simulink model "V2x_TC_IntelligentTrafficSigns_cs"  (Simulink 7.6)
settings: Solver=FixedStepDiscrete  SolverMode=SingleTasking  StartTime=0.0  StopTime=66  FixedStep=1/100  RelTol=1e-3
PreLoadFcn:
  | check_NUTS_defined
  | 
  | assignin('base',['model_closed_' bdroot],false);addpath('../includes_V2x');
InitFcn:
  | PreScanExperimentInitFcn(bdroot)
StopFcn:
  | PreScanExperimentStopFcn(bdroot);
  | 
undefined symbols (not defined in the file or in its folder): acceleration, actorID, actorType, DiscIntNeverNeededParam, GPS_alt, GPS_lat, GPS_long, lightBarInUse, messageID, Newton, publicActorType, Raphson, rotZ, sigbuilder_block, sireneInUse, trafficLightPriority, turnAdvice, velocity, x_ini_VRML, y_ini_VRML, yawRate, z_ini_VRML
libraries: simulink x111, PreScanSimulinkLibrary x7, simulink_extras x4, PreScanUsefulBlocks x3
(part 2 of 16)

== system V2x_TC_IntelligentTrafficSigns_cs/CameraTrolley ==
  SELF_CameraTrolley : Inport  Tag=SELFInport
  Dynamics_Empty : Subsystem  Tag=Dynamics_Empty
      mask Display: port_label('output', 1, 'State');text(0,0,' Empty\n')
  SELF_Demux : Subsystem  Tag=DemuxSELF
      settings: DeleteFcn=PreScanBlockDeleteFcn;
      mask Type: PreScan Demux Block:
      mask Display:
      | port_label('output',1,'x [m]')
      | port_label('output',2,'y [m]')
      | port_label('output',3,'z [m]','texmode','on')
      | port_label('output',4,'Rotx [deg]','texmode','on')
      | port_label('output',5,'Roty [deg]','texmode','on')
      | port_label('output',6,'Rotz [deg]','texmode','on')
      | port_label('output',7,'GPS lat [deg]','texmode','on')
      | port_label('output',8,'GPS long [deg]','texmode','on')
      | port_label('output',9,'GPS alt [m]','texmode','on')
      | port_label('output',10,'Velocity [m/s]')
      | port_label('output',11,'Heading [deg]')
      | port_label('output',12,'Yaw rate [deg/s]')
  Terminator_{1..13} (13 alike) : Terminator
  transmits only position : Gain  Gain=[1 1 1 0 0 0 0 0 1]
  STATE_CameraTrolley : Outport  Tag=StateOutport
  wires:
  [State_BMW] -> transmits only position/1
  SELF_CameraTrolley/1 -> SELF_Demux/1(SELF)
  transmits only position/1 -> STATE_CameraTrolley/1
  Dynamics_Empty/1(State) -> Terminator_1/1
  SELF_Demux/1(x [m]) -> Terminator_2/1
  SELF_Demux/2(y [m]) -> Terminator_3/1
  SELF_Demux/3(z [m]) -> Terminator_4/1
  SELF_Demux/4(rotx [deg]) -> Terminator_5/1
  SELF_Demux/5(roty [deg]) -> Terminator_6/1
  SELF_Demux/6(rotz [deg]) -> Terminator_7/1
  SELF_Demux/7(GPS lat [deg]) -> Terminator_8/1
  SELF_Demux/8(GPS long [deg]) -> Terminator_9/1
  SELF_Demux/9(GPS alt [m]) -> Terminator_10/1
  SELF_Demux/10(velocity [m/s]) -> Terminator_11/1
  SELF_Demux/11(heading [deg]) -> Terminator_12/1
  SELF_Demux/12(yaw rate [deg/s]) -> Terminator_13/1

== system V2x_TC_IntelligentTrafficSigns_cs/Car_Rx_1 ==
  Selector : Selector  IndexMode=Zero-based  IndexOptions=Starting index (dialog)  Indices=PortIndex  OutputSizes=PortWidth(1)
  U : Outport
  wires:
  Selector/1 -> U/1
  [SimCoreSensors] -> Selector/1

== system V2x_TC_IntelligentTrafficSigns_cs/Car_Tx_1 ==
  Y : Inport
  Assignment : Assignment  IndexMode=Zero-based  SampleTime=TimeStep  IndexOptions=Starting index (dialog)  Indices=PortIndex  OutputSizes=[1 1]
  Data Store Read : DataStoreRead  DataStoreName=SimCoreActuators  SampleTime=TimeStep
  Data Store Write : DataStoreWrite  DataStoreName=SimCoreActuators
  wires:
  Assignment/1 -> Data Store Write/1
  Data Store Read/1 -> Assignment/1
  Y/1 -> Assignment/2

== system V2x_TC_IntelligentTrafficSigns_cs/GetSensors ==
  dummy : Inport
  GetSensors : M-S-Function  FunctionName=GetSensorsBlock  Parameters=TimeStep NrOfSensors
  tag scope: SimCoreSensors=global
  wires:
  GetSensors/1 -> [SimCoreSensors]
  dummy/1 -> GetSensors/1

== system V2x_TC_IntelligentTrafficSigns_cs/Noise generator ==
  DISPLAY : M-S-Function  FunctionName=msfcn_V2X_PlotSpeed
  Scope1 : Scope  NumInputPorts=4
      settings: YMin=-5~-5~-5~-5  YMax=5~5~5~5
  tag scope: NMM_Noise=global, Tdelay=global
  wires:
  DISPLAY/1 -> Scope1/1
  DISPLAY/2 -> Scope1/2
  DISPLAY/3 -> Scope1/3, [NMM_Noise]
  DISPLAY/4 -> Scope1/4, [Tdelay]

== system V2x_TC_IntelligentTrafficSigns_cs/SELF_BMW_X5_1 ==
  Selector : Selector  IndexMode=Zero-based  IndexOptions=Starting index (dialog)  Indices=PortIndex  OutputSizes=PortWidth(1)
  U : Outport
  wires:
  Selector/1 -> U/1
  [SimCoreSensors] -> Selector/1

== system V2x_TC_IntelligentTrafficSigns_cs/SELF_CameraTrolley ==
  Selector : Selector  IndexMode=Zero-based  IndexOptions=Starting index (dialog)  Indices=PortIndex  OutputSizes=PortWidth(1)
  U : Outport
  wires:
  Selector/1 -> U/1
  [SimCoreSensors] -> Selector/1

== system V2x_TC_IntelligentTrafficSigns_cs/STATE_BMW_X5_1 ==
  State : Inport  SampleTime=TimeStep
  Acceleration : Subsystem  mask(ToSensorWorld){PortName=Acceleration_BMW_X5_1, PortIndex=6, PortWidth=3, TimeStep=1/100}
      mask prompts: PortName "Port Name [-]"; PortIndex "Index [-]"; PortWidth "Port Dimensions [-]"; TimeStep "Upload Period [s]"
      mask help: Sends signal(s) from Simulink to the Sensor World
      mask Help:
      | Sends signal(s) from Simulink to the Sensor World.
      | 
      | - Port Name [-]: specifies the name of the output terminal of the Simulink block.
      | - Index [-]: specifies the start position in the output vector sent to the Sensor World
      | - Port dimensions [-]: specifies the width (number of scalars) of the output port
      | - Upload period [s]: specifies the upload period (in seconds) of the port.
      mask Display: port_label('input', 1, PortName)
  Demux : Demux  Outputs=5
  Position : Subsystem  mask(ToSensorWorld){PortName=Position_BMW_X5_1, PortIndex=0, PortWidth=3, TimeStep=1/100}
      mask prompts: PortName "Port Name [-]"; PortIndex "Index [-]"; PortWidth "Port Dimensions [-]"; TimeStep "Upload Period [s]"
      mask help: Sends signal(s) from Simulink to the Sensor World
      mask Help:
      | Sends signal(s) from Simulink to the Sensor World.
      | 
      | - Port Name [-]: specifies the name of the output terminal of the Simulink block.
      | - Index [-]: specifies the start position in the output vector sent to the Sensor World
      | - Port dimensions [-]: specifies the width (number of scalars) of the output port
      | - Upload period [s]: specifies the upload period (in seconds) of the port.
      mask Display: port_label('input', 1, PortName)
  RPY : Subsystem  mask(ToSensorWorld){PortName=RPY_BMW_X5_1, PortIndex=9, PortWidth=3, TimeStep=1/100}
      mask prompts: PortName "Port Name [-]"; PortIndex "Index [-]"; PortWidth "Port Dimensions [-]"; TimeStep "Upload Period [s]"
      mask help: Sends signal(s) from Simulink to the Sensor World
      mask Help:
      | Sends signal(s) from Simulink to the Sensor World.
      | 
      | - Port Name [-]: specifies the name of the output terminal of the Simulink block.
      | - Index [-]: specifies the start position in the output vector sent to the Sensor World
      | - Port dimensions [-]: specifies the width (number of scalars) of the output port
      | - Upload period [s]: specifies the upload period (in seconds) of the port.
      mask Display: port_label('input', 1, PortName)
  Velocity : Subsystem  mask(ToSensorWorld){PortName=Velocity_BMW_X5_1, PortIndex=3, PortWidth=3, TimeStep=1/100}
      mask prompts: PortName "Port Name [-]"; PortIndex "Index [-]"; PortWidth "Port Dimensions [-]"; TimeStep "Upload Period [s]"
      mask help: Sends signal(s) from Simulink to the Sensor World
      mask Help:
      | Sends signal(s) from Simulink to the Sensor World.
      | 
      | - Port Name [-]: specifies the name of the output terminal of the Simulink block.
      | - Index [-]: specifies the start position in the output vector sent to the Sensor World
      | - Port dimensions [-]: specifies the width (number of scalars) of the output port
      | - Upload period [s]: specifies the upload period (in seconds) of the port.
      mask Display: port_label('input', 1, PortName)
  iniBODYAngles wrtVEH [rad] : Constant  Value=[Roll0 Pitch0 0]  SampleTime=TimeStep
  iniVEHAngles wrtGLOB [rad] : Constant  Value=[0 0 Yaw0]  SampleTime=TimeStep
  iniVEHposition wrtGLOB [m] : Constant  Value=[x0 y0 z0]  SampleTime=TimeStep
  state2FullState : PreScanSimulinkLibrary/state2FullState  SystemSampleTime=-1
  velRPY : Subsystem  mask(ToSensorWorld){PortName=velRPY_BMW_X5_1, PortIndex=12, PortWidth=3, TimeStep=1/100}
      mask prompts: PortName "Port Name [-]"; PortIndex "Index [-]"; PortWidth "Port Dimensions [-]"; TimeStep "Upload Period [s]"
      mask help: Sends signal(s) from Simulink to the Sensor World
      mask Help:
      | Sends signal(s) from Simulink to the Sensor World.
      | 
      | - Port Name [-]: specifies the name of the output terminal of the Simulink block.
      | - Index [-]: specifies the start position in the output vector sent to the Sensor World
      | - Port dimensions [-]: specifies the width (number of scalars) of the output port
      | - Upload period [s]: specifies the upload period (in seconds) of the port.
      mask Display: port_label('input', 1, PortName)
  wires:
  Demux/5 -> velRPY/1(Y)
  Demux/4 -> RPY/1(Y)
  Demux/3 -> Acceleration/1(Y)
  Demux/2 -> Velocity/1(Y)
  Demux/1 -> Position/1(Y)
  State/1 -> state2FullState/1
  state2FullState/1 -> Demux/1
  iniVEHposition wrtGLOB [m]/1 -> state2FullState/2
  iniBODYAngles wrtVEH [rad]/1 -> state2FullState/3
  iniVEHAngles wrtGLOB [rad]/1 -> state2FullState/4

== system V2x_TC_IntelligentTrafficSigns_cs/STATE_CameraTrolley ==
  State : Inport  SampleTime=TimeStep
  Acceleration : Subsystem  mask(ToSensorWorld){PortName=Acceleration_CameraTrolley, PortIndex=454, PortWidth=3, TimeStep=1/100}
      mask prompts: PortName "Port Name [-]"; PortIndex "Index [-]"; PortWidth "Port Dimensions [-]"; TimeStep "Upload Period [s]"
      mask help: Sends signal(s) from Simulink to the Sensor World
      mask Help:
      | Sends signal(s) from Simulink to the Sensor World.
      | 
      | - Port Name [-]: specifies the name of the output terminal of the Simulink block.
      | - Index [-]: specifies the start position in the output vector sent to the Sensor World
      | - Port dimensions [-]: specifies the width (number of scalars) of the output port
      | - Upload period [s]: specifies the upload period (in seconds) of the port.
      mask Display: port_label('input', 1, PortName)
  Demux : Demux  Outputs=5
  Position : Subsystem  mask(ToSensorWorld){PortName=Position_CameraTrolley, PortIndex=448, PortWidth=3, TimeStep=1/100}
      mask prompts: PortName "Port Name [-]"; PortIndex "Index [-]"; PortWidth "Port Dimensions [-]"; TimeStep "Upload Period [s]"
      mask help: Sends signal(s) from Simulink to the Sensor World
      mask Help:
      | Sends signal(s) from Simulink to the Sensor World.
      | 
      | - Port Name [-]: specifies the name of the output terminal of the Simulink block.
      | - Index [-]: specifies the start position in the output vector sent to the Sensor World
      | - Port dimensions [-]: specifies the width (number of scalars) of the output port
      | - Upload period [s]: specifies the upload period (in seconds) of the port.
      mask Display: port_label('input', 1, PortName)
  RPY : Subsystem  mask(ToSensorWorld){PortName=RPY_CameraTrolley, PortIndex=457, PortWidth=3, TimeStep=1/100}
      mask prompts: PortName "Port Name [-]"; PortIndex "Index [-]"; PortWidth "Port Dimensions [-]"; TimeStep "Upload Period [s]"
      mask help: Sends signal(s) from Simulink to the Sensor World
      mask Help:
      | Sends signal(s) from Simulink to the Sensor World.
      | 
      | - Port Name [-]: specifies the name of the output terminal of the Simulink block.
      | - Index [-]: specifies the start position in the output vector sent to the Sensor World
      | - Port dimensions [-]: specifies the width (number of scalars) of the output port
      | - Upload period [s]: specifies the upload period (in seconds) of the port.
      mask Display: port_label('input', 1, PortName)
  Velocity : Subsystem  mask(ToSensorWorld){PortName=Velocity_CameraTrolley, PortIndex=451, PortWidth=3, TimeStep=1/100}
      mask prompts: PortName "Port Name [-]"; PortIndex "Index [-]"; PortWidth "Port Dimensions [-]"; TimeStep "Upload Period [s]"
      mask help: Sends signal(s) from Simulink to the Sensor World
      mask Help:
      | Sends signal(s) from Simulink to the Sensor World.
      | 
      | - Port Name [-]: specifies the name of the output terminal of the Simulink block.
      | - Index [-]: specifies the start position in the output vector sent to the Sensor World
      | - Port dimensions [-]: specifies the width (number of scalars) of the output port
      | - Upload period [s]: specifies the upload period (in seconds) of the port.
      mask Display: port_label('input', 1, PortName)
  iniBODYAngles wrtVEH [rad] : Constant  Value=[Roll0 Pitch0 0]  SampleTime=TimeStep
  iniVEHAngles wrtGLOB [rad] : Constant  Value=[0 0 Yaw0]  SampleTime=TimeStep
  iniVEHposition wrtGLOB [m] : Constant  Value=[x0 y0 z0]  SampleTime=TimeStep
  state2FullState : PreScanSimulinkLibrary/state2FullState  SystemSampleTime=-1
  velRPY : Subsystem  mask(ToSensorWorld){PortName=velRPY_CameraTrolley, PortIndex=460, PortWidth=3, TimeStep=1/100}
      mask prompts: PortName "Port Name [-]"; PortIndex "Index [-]"; PortWidth "Port Dimensions [-]"; TimeStep "Upload Period [s]"
      mask help: Sends signal(s) from Simulink to the Sensor World
      mask Help:
      | Sends signal(s) from Simulink to the Sensor World.
      | 
      | - Port Name [-]: specifies the name of the output terminal of the Simulink block.
      | - Index [-]: specifies the start position in the output vector sent to the Sensor World
      | - Port dimensions [-]: specifies the width (number of scalars) of the output port
      | - Upload period [s]: specifies the upload period (in seconds) of the port.
      mask Display: port_label('input', 1, PortName)
  wires:
  Demux/5 -> velRPY/1(Y)
  Demux/4 -> RPY/1(Y)
  Demux/3 -> Acceleration/1(Y)
  Demux/2 -> Velocity/1(Y)
  Demux/1 -> Position/1(Y)
  State/1 -> state2FullState/1
  state2FullState/1 -> Demux/1
  iniVEHposition wrtGLOB [m]/1 -> state2FullState/2
  iniBODYAngles wrtVEH [rad]/1 -> state2FullState/3
  iniVEHAngles wrtGLOB [rad]/1 -> state2FullState/4

== system V2x_TC_IntelligentTrafficSigns_cs/SetActuators ==
  Bus Creator : BusCreator  Inputs=1
  Data Store Read : DataStoreRead  DataStoreName=SimCoreActuators  SampleTime=TimeStep
  SendActuators : M-S-Function  FunctionName=SendActuatorsBlock  Parameters=TimeStep NrOfActuators
  dummy : Outport
  tag scope: SimCoreInfo=global
  wires:
  Bus Creator/1 -> [SimCoreInfo]  "SimCore"
  SendActuators/2 -> Bus Creator/1  "ComputationTime[s]"
  SendActuators/1 -> dummy/1
  Data Store Read/1 -> SendActuators/1

== system V2x_TC_IntelligentTrafficSigns_cs/SimCoreInfo ==
  Bus Selector : BusSelector  OutputSignals=ComputationTime[s]
  Time : Scope
      settings: TimeRange=10  YMin=0  YMax=0.5  SaveName=ComputationTime
  wires:
  Bus Selector/1 -> Time/1  "<ComputationTime[s]>"
  [SimCoreInfo] -> Bus Selector/1

== system V2x_TC_IntelligentTrafficSigns_cs/TS_Lcurve_R14 ==
  CAM generation time step : Constant  Value=0.1
  CAM repetition time step : Constant  Value=0.05
  Cartesian to GPS : Subsystem
  Green light : Constant  Value=0
  Ground_{1..5} (5 alike) : Ground
  Infrastructure CAM generator : Subsystem
  Orange light : Constant  Value=0
  Red light : Constant  Value=0
  Tx_Lcurve_R14_BusAssignment : BusAssignment  Tag=BusAntennaT  AssignedSignals=warning,messageID,actorID,actorType,trafficLightPriority,GPS_lat,GPS_long,GPS_alt,rotZ
  Tx_Lcurve_R14_Mux : Subsystem  Tag=MuxAntennaT  mask(PreScan Mux Block:){Channel=1, TimeStep=1/100}
      mask prompts: TimeStep "Time Step [s]"
      settings: DeleteFcn=PreScanBlockDeleteFcn;
      mask Display:
      | fprintf('Channel: %d',Channel)
      | port_label('input', 1, 'Warning signal ID')
      | port_label('input', 2, 'Maneuvre ID')
      | port_label('input', 3, 'Vehicle Type')
      | port_label('input', 4, 'Braking Capacity')
      | port_label('input', 5, 'Vehicle Size')
      | port_label('input', 6, 'spare')
  X : Constant  Value=-192.331085
  Y : Constant  Value=-63.62283
  Z : Constant  Value=0
  actorID : Constant  Value=32
  actorType : Constant  Value=14
  messageID : Constant  Value=902
  rotZ : Constant  Value=90
  warning : Constant  Value=0
  Tx_Lcurve_R14 : Outport  Tag=AntennaTOutport
  wires:
  CAM generation time step/1 -> Infrastructure CAM generator/1(CAM gen time step)
  CAM repetition time step/1 -> Infrastructure CAM generator/2(CAM rep time step)
  warning/1 -> Infrastructure CAM generator/3(warning)
  messageID/1 -> Infrastructure CAM generator/4(messageID)
  actorID/1 -> Infrastructure CAM generator/5(actorID)
  actorType/1 -> Infrastructure CAM generator/6(actorType)
  Red light/1 -> Infrastructure CAM generator/7(Red light)
  Orange light/1 -> Infrastructure CAM generator/8(Orange light)
  Green light/1 -> Infrastructure CAM generator/9(Green light)
  rotZ/1 -> Infrastructure CAM generator/13(rotZ)
  X/1 -> Cartesian to GPS/1(X)
  Y/1 -> Cartesian to GPS/2(Y)
  Z/1 -> Cartesian to GPS/3(Z)
  Cartesian to GPS/1(lat) -> Infrastructure CAM generator/10(GPS_lat)
  Cartesian to GPS/2(long) -> Infrastructure CAM generator/11(GPS_long)
  Cartesian to GPS/3(alt) -> Infrastructure CAM generator/12(GPS_alt)
  Tx_Lcurve_R14_Mux/2(Out2) -> [Tx_Lcurve_R14]
  [Tx_Lcurve_R14] -> Tx_Lcurve_R14_BusAssignment/1
  Tx_Lcurve_R14_BusAssignment/1 -> Tx_Lcurve_R14_Mux/6(spare)
  Tx_Lcurve_R14_Mux/1(Out1) -> Tx_Lcurve_R14/1
  Infrastructure CAM generator/9(CAM_rotZ) -> Tx_Lcurve_R14_BusAssignment/10
  Infrastructure CAM generator/8(CAM_GPSalt) -> Tx_Lcurve_R14_BusAssignment/9
  Infrastructure CAM generator/7(CAM_GPSlong) -> Tx_Lcurve_R14_BusAssignment/8
  Infrastructure CAM generator/6(CAM_GPSlat) -> Tx_Lcurve_R14_BusAssignment/7
  Infrastructure CAM generator/5(CAM_trafficLightPriority) -> Tx_Lcurve_R14_BusAssignment/6
  Infrastructure CAM generator/4(CAM_vehicleType) -> Tx_Lcurve_R14_BusAssignment/5
  Infrastructure CAM generator/3(CAM_vehicleID) -> Tx_Lcurve_R14_BusAssignment/4
  Infrastructure CAM generator/2(CAM_messageID) -> Tx_Lcurve_R14_BusAssignment/3
  Infrastructure CAM generator/1(CAM_warning) -> Tx_Lcurve_R14_BusAssignment/2
  Ground_1/1 -> Tx_Lcurve_R14_Mux/1(warning_signal_id)
  Ground_2/1 -> Tx_Lcurve_R14_Mux/2(maneuvre_id)
  Ground_3/1 -> Tx_Lcurve_R14_Mux/3(vehicle_type)
  Ground_4/1 -> Tx_Lcurve_R14_Mux/4(brakingcapacity)
  Ground_5/1 -> Tx_Lcurve_R14_Mux/5(vehicle_size)

== system V2x_TC_IntelligentTrafficSigns_cs/TS_Lcurve_R25 ==
  CAM generation time step : Constant  Value=0.1
  CAM repetition time step : Constant  Value=0.05
  Cartesian to GPS : Subsystem
  Green light : Constant  Value=0
  Ground_{1..5} (5 alike) : Ground
  Infrastructure CAM generator : Subsystem
  Orange light : Constant  Value=0
  Red light : Constant  Value=0
  Tx_Lcurve_R25_BusAssignment : BusAssignment  Tag=BusAntennaT  AssignedSignals=warning,messageID,actorID,actorType,trafficLightPriority,GPS_lat,GPS_long,GPS_alt,rotZ
  Tx_Lcurve_R25_Mux : Subsystem  Tag=MuxAntennaT  mask(PreScan Mux Block:){Channel=1, TimeStep=1/100}
      mask prompts: TimeStep "Time Step [s]"
      settings: DeleteFcn=PreScanBlockDeleteFcn;
      mask Display:
      | fprintf('Channel: %d',Channel)
      | port_label('input', 1, 'Warning signal ID')
      | port_label('input', 2, 'Maneuvre ID')
      | port_label('input', 3, 'Vehicle Type')
      | port_label('input', 4, 'Braking Capacity')
      | port_label('input', 5, 'Vehicle Size')
      | port_label('input', 6, 'spare')
  X : Constant  Value=-105.98568
  Y : Constant  Value=-53.2315674
  Z : Constant  Value=0
  actorID : Constant  Value=902
  actorType : Constant  Value=14
  messageID : Constant  Value=902
  rotZ : Constant  Value=280
  warning : Constant  Value=0
  Tx_Lcurve_R25 : Outport  Tag=AntennaTOutport
  wires:
  CAM generation time step/1 -> Infrastructure CAM generator/1(CAM gen time step)
  CAM repetition time step/1 -> Infrastructure CAM generator/2(CAM rep time step)
  warning/1 -> Infrastructure CAM generator/3(warning)
  messageID/1 -> Infrastructure CAM generator/4(messageID)
  actorID/1 -> Infrastructure CAM generator/5(actorID)
  actorType/1 -> Infrastructure CAM generator/6(actorType)
  Red light/1 -> Infrastructure CAM generator/7(Red light)
  Orange light/1 -> Infrastructure CAM generator/8(Orange light)
  Green light/1 -> Infrastructure CAM generator/9(Green light)
  rotZ/1 -> Infrastructure CAM generator/13(rotZ)
  X/1 -> Cartesian to GPS/1(X)
  Y/1 -> Cartesian to GPS/2(Y)
  Z/1 -> Cartesian to GPS/3(Z)
  Cartesian to GPS/1(lat) -> Infrastructure CAM generator/10(GPS_lat)
  Cartesian to GPS/2(long) -> Infrastructure CAM generator/11(GPS_long)
  Cartesian to GPS/3(alt) -> Infrastructure CAM generator/12(GPS_alt)
  Tx_Lcurve_R25_Mux/2(Out2) -> [Tx_Lcurve_R25]
  [Tx_Lcurve_R25] -> Tx_Lcurve_R25_BusAssignment/1
  Tx_Lcurve_R25_BusAssignment/1 -> Tx_Lcurve_R25_Mux/6(spare)
  Tx_Lcurve_R25_Mux/1(Out1) -> Tx_Lcurve_R25/1
  Infrastructure CAM generator/9(CAM_rotZ) -> Tx_Lcurve_R25_BusAssignment/10
  Infrastructure CAM generator/8(CAM_GPSalt) -> Tx_Lcurve_R25_BusAssignment/9
  Infrastructure CAM generator/7(CAM_GPSlong) -> Tx_Lcurve_R25_BusAssignment/8
  Infrastructure CAM generator/6(CAM_GPSlat) -> Tx_Lcurve_R25_BusAssignment/7
  Infrastructure CAM generator/5(CAM_trafficLightPriority) -> Tx_Lcurve_R25_BusAssignment/6
  Infrastructure CAM generator/4(CAM_vehicleType) -> Tx_Lcurve_R25_BusAssignment/5
  Infrastructure CAM generator/3(CAM_vehicleID) -> Tx_Lcurve_R25_BusAssignment/4
  Infrastructure CAM generator/2(CAM_messageID) -> Tx_Lcurve_R25_BusAssignment/3
  Infrastructure CAM generator/1(CAM_warning) -> Tx_Lcurve_R25_BusAssignment/2
  Ground_1/1 -> Tx_Lcurve_R25_Mux/1(warning_signal_id)
  Ground_2/1 -> Tx_Lcurve_R25_Mux/2(maneuvre_id)
  Ground_3/1 -> Tx_Lcurve_R25_Mux/3(vehicle_type)
  Ground_4/1 -> Tx_Lcurve_R25_Mux/4(brakingcapacity)
  Ground_5/1 -> Tx_Lcurve_R25_Mux/5(vehicle_size)

== system V2x_TC_IntelligentTrafficSigns_cs/TS_Lcurve_R39 ==
  CAM generation time step : Constant  Value=0.1
  CAM repetition time step : Constant  Value=0.05
  Cartesian to GPS : Subsystem
  Green light : Constant  Value=0
  Ground_{1..5} (5 alike) : Ground
  Infrastructure CAM generator : Subsystem
  Orange light : Constant  Value=0
  Red light : Constant  Value=0
  Tx_Lcurve_R39_BusAssignment : BusAssignment  Tag=BusAntennaT  AssignedSignals=warning,messageID,actorID,actorType,trafficLightPriority,GPS_lat,GPS_long,GPS_alt,rotZ
  Tx_Lcurve_R39_Mux : Subsystem  Tag=MuxAntennaT  mask(PreScan Mux Block:){Channel=1, TimeStep=1/100}
      mask prompts: TimeStep "Time Step [s]"
      settings: DeleteFcn=PreScanBlockDeleteFcn;
      mask Display:
      | fprintf('Channel: %d',Channel)
      | port_label('input', 1, 'Warning signal ID')
      | port_label('input', 2, 'Maneuvre ID')
      | port_label('input', 3, 'Vehicle Type')
      | port_label('input', 4, 'Braking Capacity')
      | port_label('input', 5, 'Vehicle Size')
      | port_label('input', 6, 'spare')
  X : Constant  Value=-115.560738
  Y : Constant  Value=-55.21178
  Z : Constant  Value=0
  actorID : Constant  Value=29
  actorType : Constant  Value=14
  messageID : Constant  Value=902
  rotZ : Constant  Value=100
  warning : Constant  Value=0
  Tx_Lcurve_R39 : Outport  Tag=AntennaTOutport
  wires:
  CAM generation time step/1 -> Infrastructure CAM generator/1(CAM gen time step)
  CAM repetition time step/1 -> Infrastructure CAM generator/2(CAM rep time step)
  warning/1 -> Infrastructure CAM generator/3(warning)
  messageID/1 -> Infrastructure CAM generator/4(messageID)
  actorID/1 -> Infrastructure CAM generator/5(actorID)
  actorType/1 -> Infrastructure CAM generator/6(actorType)
  Red light/1 -> Infrastructure CAM generator/7(Red light)
  Orange light/1 -> Infrastructure CAM generator/8(Orange light)
  Green light/1 -> Infrastructure CAM generator/9(Green light)
  rotZ/1 -> Infrastructure CAM generator/13(rotZ)
  X/1 -> Cartesian to GPS/1(X)
  Y/1 -> Cartesian to GPS/2(Y)
  Z/1 -> Cartesian to GPS/3(Z)
  Cartesian to GPS/1(lat) -> Infrastructure CAM generator/10(GPS_lat)
  Cartesian to GPS/2(long) -> Infrastructure CAM generator/11(GPS_long)
  Cartesian to GPS/3(alt) -> Infrastructure CAM generator/12(GPS_alt)
  Tx_Lcurve_R39_Mux/2(Out2) -> [Tx_Lcurve_R39]
  [Tx_Lcurve_R39] -> Tx_Lcurve_R39_BusAssignment/1
  Tx_Lcurve_R39_BusAssignment/1 -> Tx_Lcurve_R39_Mux/6(spare)
  Tx_Lcurve_R39_Mux/1(Out1) -> Tx_Lcurve_R39/1
  Infrastructure CAM generator/9(CAM_rotZ) -> Tx_Lcurve_R39_BusAssignment/10
  Infrastructure CAM generator/8(CAM_GPSalt) -> Tx_Lcurve_R39_BusAssignment/9
  Infrastructure CAM generator/7(CAM_GPSlong) -> Tx_Lcurve_R39_BusAssignment/8
  Infrastructure CAM generator/6(CAM_GPSlat) -> Tx_Lcurve_R39_BusAssignment/7
  Infrastructure CAM generator/5(CAM_trafficLightPriority) -> Tx_Lcurve_R39_BusAssignment/6
  Infrastructure CAM generator/4(CAM_vehicleType) -> Tx_Lcurve_R39_BusAssignment/5
  Infrastructure CAM generator/3(CAM_vehicleID) -> Tx_Lcurve_R39_BusAssignment/4
  Infrastructure CAM generator/2(CAM_messageID) -> Tx_Lcurve_R39_BusAssignment/3
  Infrastructure CAM generator/1(CAM_warning) -> Tx_Lcurve_R39_BusAssignment/2
  Ground_1/1 -> Tx_Lcurve_R39_Mux/1(warning_signal_id)
  Ground_2/1 -> Tx_Lcurve_R39_Mux/2(maneuvre_id)
  Ground_3/1 -> Tx_Lcurve_R39_Mux/3(vehicle_type)
  Ground_4/1 -> Tx_Lcurve_R39_Mux/4(brakingcapacity)
  Ground_5/1 -> Tx_Lcurve_R39_Mux/5(vehicle_size)

== system V2x_TC_IntelligentTrafficSigns_cs/TS_Lcurve_R55 ==
  CAM generation time step : Constant  Value=0.1
  CAM repetition time step : Constant  Value=0.05
  Cartesian to GPS : Subsystem
  Green light : Constant  Value=0
  Ground_{1..5} (5 alike) : Ground
  Infrastructure CAM generator : Subsystem
  Orange light : Constant  Value=0
  Red light : Constant  Value=0
  Tx_Lcurve_R55_BusAssignment : BusAssignment  Tag=BusAntennaT  AssignedSignals=warning,messageID,actorID,actorType,trafficLightPriority,GPS_lat,GPS_long,GPS_alt,rotZ
  Tx_Lcurve_R55_Mux : Subsystem  Tag=MuxAntennaT  mask(PreScan Mux Block:){Channel=1, TimeStep=1/100}
      mask prompts: TimeStep "Time Step [s]"
      settings: DeleteFcn=PreScanBlockDeleteFcn;
      mask Display:
      | fprintf('Channel: %d',Channel)
      | port_label('input', 1, 'Warning signal ID')
      | port_label('input', 2, 'Maneuvre ID')
      | port_label('input', 3, 'Vehicle Type')
      | port_label('input', 4, 'Braking Capacity')
      | port_label('input', 5, 'Vehicle Size')
      | port_label('input', 6, 'spare')
  X : Constant  Value=-13.9763317
  Y : Constant  Value=48.9121056
  Z : Constant  Value=0
  actorID : Constant  Value=23
  actorType : Constant  Value=14
  messageID : Constant  Value=902
  rotZ : Constant  Value=270
  warning : Constant  Value=0
  Tx_Lcurve_R55 : Outport  Tag=AntennaTOutport
  wires:
  CAM generation time step/1 -> Infrastructure CAM generator/1(CAM gen time step)
  CAM repetition time step/1 -> Infrastructure CAM generator/2(CAM rep time step)
  warning/1 -> Infrastructure CAM generator/3(warning)
  messageID/1 -> Infrastructure CAM generator/4(messageID)
  actorID/1 -> Infrastructure CAM generator/5(actorID)
  actorType/1 -> Infrastructure CAM generator/6(actorType)
  Red light/1 -> Infrastructure CAM generator/7(Red light)
  Orange light/1 -> Infrastructure CAM generator/8(Orange light)
  Green light/1 -> Infrastructure CAM generator/9(Green light)
  rotZ/1 -> Infrastructure CAM generator/13(rotZ)
  X/1 -> Cartesian to GPS/1(X)
  Y/1 -> Cartesian to GPS/2(Y)
  Z/1 -> Cartesian to GPS/3(Z)
  Cartesian to GPS/1(lat) -> Infrastructure CAM generator/10(GPS_lat)
  Cartesian to GPS/2(long) -> Infrastructure CAM generator/11(GPS_long)
  Cartesian to GPS/3(alt) -> Infrastructure CAM generator/12(GPS_alt)
  Tx_Lcurve_R55_Mux/2(Out2) -> [Tx_Lcurve_R55]
  [Tx_Lcurve_R55] -> Tx_Lcurve_R55_BusAssignment/1
  Tx_Lcurve_R55_BusAssignment/1 -> Tx_Lcurve_R55_Mux/6(spare)
  Tx_Lcurve_R55_Mux/1(Out1) -> Tx_Lcurve_R55/1
  Infrastructure CAM generator/9(CAM_rotZ) -> Tx_Lcurve_R55_BusAssignment/10
  Infrastructure CAM generator/8(CAM_GPSalt) -> Tx_Lcurve_R55_BusAssignment/9
  Infrastructure CAM generator/7(CAM_GPSlong) -> Tx_Lcurve_R55_BusAssignment/8
  Infrastructure CAM generator/6(CAM_GPSlat) -> Tx_Lcurve_R55_BusAssignment/7
  Infrastructure CAM generator/5(CAM_trafficLightPriority) -> Tx_Lcurve_R55_BusAssignment/6
  Infrastructure CAM generator/4(CAM_vehicleType) -> Tx_Lcurve_R55_BusAssignment/5
  Infrastructure CAM generator/3(CAM_vehicleID) -> Tx_Lcurve_R55_BusAssignment/4
  Infrastructure CAM generator/2(CAM_messageID) -> Tx_Lcurve_R55_BusAssignment/3
  Infrastructure CAM generator/1(CAM_warning) -> Tx_Lcurve_R55_BusAssignment/2
  Ground_1/1 -> Tx_Lcurve_R55_Mux/1(warning_signal_id)
  Ground_2/1 -> Tx_Lcurve_R55_Mux/2(maneuvre_id)
  Ground_3/1 -> Tx_Lcurve_R55_Mux/3(vehicle_type)
  Ground_4/1 -> Tx_Lcurve_R55_Mux/4(brakingcapacity)
  Ground_5/1 -> Tx_Lcurve_R55_Mux/5(vehicle_size)

== system V2x_TC_IntelligentTrafficSigns_cs/TS_Rcurve_R14 ==
  CAM generation time step : Constant  Value=0.1
  CAM repetition time step : Constant  Value=0.05
  Cartesian to GPS : Subsystem
  Green light : Constant  Value=0
  Ground_{1..5} (5 alike) : Ground
  Infrastructure CAM generator : Subsystem
  Orange light : Constant  Value=0
  Red light : Constant  Value=0
  Tx_Rcurve_R14_BusAssignment : BusAssignment  Tag=BusAntennaT  AssignedSignals=warning,messageID,actorID,actorType,trafficLightPriority,GPS_lat,GPS_long,GPS_alt,rotZ
  Tx_Rcurve_R14_Mux : Subsystem  Tag=MuxAntennaT  mask(PreScan Mux Block:){Channel=1, TimeStep=1/100}
      mask prompts: TimeStep "Time Step [s]"
      settings: DeleteFcn=PreScanBlockDeleteFcn;
      mask Display:
      | fprintf('Channel: %d',Channel)
      | port_label('input', 1, 'Warning signal ID')
      | port_label('input', 2, 'Maneuvre ID')
      | port_label('input', 3, 'Vehicle Type')
      | port_label('input', 4, 'Braking Capacity')
      | port_label('input', 5, 'Vehicle Size')
      | port_label('input', 6, 'spare')
  X : Constant  Value=-164.078568
  Y : Constant  Value=-64.77414
  Z : Constant  Value=0
  actorID : Constant  Value=31
  actorType : Constant  Value=14
  messageID : Constant  Value=904
  rotZ : Constant  Value=95
  warning : Constant  Value=0
  Tx_Rcurve_R14 : Outport  Tag=AntennaTOutport
  wires:
  CAM generation time step/1 -> Infrastructure CAM generator/1(CAM gen time step)
  CAM repetition time step/1 -> Infrastructure CAM generator/2(CAM rep time step)
  warning/1 -> Infrastructure CAM generator/3(warning)
  messageID/1 -> Infrastructure CAM generator/4(messageID)
  actorID/1 -> Infrastructure CAM generator/5(actorID)
  actorType/1 -> Infrastructure CAM generator/6(actorType)
  Red light/1 -> Infrastructure CAM generator/7(Red light)
  Orange light/1 -> Infrastructure CAM generator/8(Orange light)
  Green light/1 -> Infrastructure CAM generator/9(Green light)
  rotZ/1 -> Infrastructure CAM generator/13(rotZ)
  X/1 -> Cartesian to GPS/1(X)
  Y/1 -> Cartesian to GPS/2(Y)
  Z/1 -> Cartesian to GPS/3(Z)
  Cartesian to GPS/1(lat) -> Infrastructure CAM generator/10(GPS_lat)
  Cartesian to GPS/2(long) -> Infrastructure CAM generator/11(GPS_long)
  Cartesian to GPS/3(alt) -> Infrastructure CAM generator/12(GPS_alt)
  Tx_Rcurve_R14_Mux/2(Out2) -> [Tx_Rcurve_R14]
  [Tx_Rcurve_R14] -> Tx_Rcurve_R14_BusAssignment/1
  Tx_Rcurve_R14_BusAssignment/1 -> Tx_Rcurve_R14_Mux/6(spare)
  Tx_Rcurve_R14_Mux/1(Out1) -> Tx_Rcurve_R14/1
  Infrastructure CAM generator/9(CAM_rotZ) -> Tx_Rcurve_R14_BusAssignment/10
  Infrastructure CAM generator/8(CAM_GPSalt) -> Tx_Rcurve_R14_BusAssignment/9
  Infrastructure CAM generator/7(CAM_GPSlong) -> Tx_Rcurve_R14_BusAssignment/8
  Infrastructure CAM generator/6(CAM_GPSlat) -> Tx_Rcurve_R14_BusAssignment/7
  Infrastructure CAM generator/5(CAM_trafficLightPriority) -> Tx_Rcurve_R14_BusAssignment/6
  Infrastructure CAM generator/4(CAM_vehicleType) -> Tx_Rcurve_R14_BusAssignment/5
  Infrastructure CAM generator/3(CAM_vehicleID) -> Tx_Rcurve_R14_BusAssignment/4
  Infrastructure CAM generator/2(CAM_messageID) -> Tx_Rcurve_R14_BusAssignment/3
  Infrastructure CAM generator/1(CAM_warning) -> Tx_Rcurve_R14_BusAssignment/2
  Ground_1/1 -> Tx_Rcurve_R14_Mux/1(warning_signal_id)
  Ground_2/1 -> Tx_Rcurve_R14_Mux/2(maneuvre_id)
  Ground_3/1 -> Tx_Rcurve_R14_Mux/3(vehicle_type)
  Ground_4/1 -> Tx_Rcurve_R14_Mux/4(brakingcapacity)
  Ground_5/1 -> Tx_Rcurve_R14_Mux/5(vehicle_size)

== system V2x_TC_IntelligentTrafficSigns_cs/TS_Rcurve_R25 ==
  CAM generation time step : Constant  Value=0.1
  CAM repetition time step : Constant  Value=0.05
  Cartesian to GPS : Subsystem
  Green light : Constant  Value=0
  Ground_{1..5} (5 alike) : Ground
  Infrastructure CAM generator : Subsystem
  Orange light : Constant  Value=0
  Red light : Constant  Value=0
  Tx_Rcurve_R25_BusAssignment : BusAssignment  Tag=BusAntennaT  AssignedSignals=warning,messageID,actorID,actorType,trafficLightPriority,GPS_lat,GPS_long,GPS_alt,rotZ
  Tx_Rcurve_R25_Mux : Subsystem  Tag=MuxAntennaT  mask(PreScan Mux Block:){Channel=1, TimeStep=1/100}
      mask prompts: TimeStep "Time Step [s]"
      settings: DeleteFcn=PreScanBlockDeleteFcn;
      mask Display:
      | fprintf('Channel: %d',Channel)
      | port_label('input', 1, 'Warning signal ID')
      | port_label('input', 2, 'Maneuvre ID')
      | port_label('input', 3, 'Vehicle Type')
      | port_label('input', 4, 'Braking Capacity')
      | port_label('input', 5, 'Vehicle Size')
      | port_label('input', 6, 'spare')
  X : Constant  Value=-154.943542
  Y : Constant  Value=-63.75283
  Z : Constant  Value=0
  actorID : Constant  Value=26
  actorType : Constant  Value=14
  messageID : Constant  Value=904
  rotZ : Constant  Value=270
  warning : Constant  Value=0
  Tx_Rcurve_R25 : Outport  Tag=AntennaTOutport
  wires:
  CAM generation time step/1 -> Infrastructure CAM generator/1(CAM gen time step)
  CAM repetition time step/1 -> Infrastructure CAM generator/2(CAM rep time step)
  warning/1 -> Infrastructure CAM generator/3(warning)
  messageID/1 -> Infrastructure CAM generator/4(messageID)
  actorID/1 -> Infrastructure CAM generator/5(actorID)
  actorType/1 -> Infrastructure CAM generator/6(actorType)
  Red light/1 -> Infrastructure CAM generator/7(Red light)
  Orange light/1 -> Infrastructure CAM generator/8(Orange light)
  Green light/1 -> Infrastructure CAM generator/9(Green light)
  rotZ/1 -> Infrastructure CAM generator/13(rotZ)
  X/1 -> Cartesian to GPS/1(X)
  Y/1 -> Cartesian to GPS/2(Y)
  Z/1 -> Cartesian to GPS/3(Z)
  Cartesian to GPS/1(lat) -> Infrastructure CAM generator/10(GPS_lat)
  Cartesian to GPS/2(long) -> Infrastructure CAM generator/11(GPS_long)
  Cartesian to GPS/3(alt) -> Infrastructure CAM generator/12(GPS_alt)
  Tx_Rcurve_R25_Mux/2(Out2) -> [Tx_Rcurve_R25]
  [Tx_Rcurve_R25] -> Tx_Rcurve_R25_BusAssignment/1
  Tx_Rcurve_R25_BusAssignment/1 -> Tx_Rcurve_R25_Mux/6(spare)
  Tx_Rcurve_R25_Mux/1(Out1) -> Tx_Rcurve_R25/1
  Infrastructure CAM generator/9(CAM_rotZ) -> Tx_Rcurve_R25_BusAssignment/10
  Infrastructure CAM generator/8(CAM_GPSalt) -> Tx_Rcurve_R25_BusAssignment/9
  Infrastructure CAM generator/7(CAM_GPSlong) -> Tx_Rcurve_R25_BusAssignment/8
  Infrastructure CAM generator/6(CAM_GPSlat) -> Tx_Rcurve_R25_BusAssignment/7
  Infrastructure CAM generator/5(CAM_trafficLightPriority) -> Tx_Rcurve_R25_BusAssignment/6
  Infrastructure CAM generator/4(CAM_vehicleType) -> Tx_Rcurve_R25_BusAssignment/5
  Infrastructure CAM generator/3(CAM_vehicleID) -> Tx_Rcurve_R25_BusAssignment/4
  Infrastructure CAM generator/2(CAM_messageID) -> Tx_Rcurve_R25_BusAssignment/3
  Infrastructure CAM generator/1(CAM_warning) -> Tx_Rcurve_R25_BusAssignment/2
  Ground_1/1 -> Tx_Rcurve_R25_Mux/1(warning_signal_id)
  Ground_2/1 -> Tx_Rcurve_R25_Mux/2(maneuvre_id)
  Ground_3/1 -> Tx_Rcurve_R25_Mux/3(vehicle_type)
  Ground_4/1 -> Tx_Rcurve_R25_Mux/4(brakingcapacity)
  Ground_5/1 -> Tx_Rcurve_R25_Mux/5(vehicle_size)

== system V2x_TC_IntelligentTrafficSigns_cs/TS_Rcurve_R39 ==
  CAM generation time step : Constant  Value=0.1
  CAM repetition time step : Constant  Value=0.05
  Cartesian to GPS : Subsystem
  Green light : Constant  Value=0
  Ground_{1..5} (5 alike) : Ground
  Infrastructure CAM generator : Subsystem
  Orange light : Constant  Value=0
  Red light : Constant  Value=0
  Tx_Rcurve_R39_BusAssignment : BusAssignment  Tag=BusAntennaT  AssignedSignals=warning,messageID,actorID,actorType,trafficLightPriority,GPS_lat,GPS_long,GPS_alt,rotZ
  Tx_Rcurve_R39_Mux : Subsystem  Tag=MuxAntennaT  mask(PreScan Mux Block:){Channel=1, TimeStep=1/100}
      mask prompts: TimeStep "Time Step [s]"
      settings: DeleteFcn=PreScanBlockDeleteFcn;
      mask Display:
      | fprintf('Channel: %d',Channel)
      | port_label('input', 1, 'Warning signal ID')
      | port_label('input', 2, 'Maneuvre ID')
      | port_label('input', 3, 'Vehicle Type')
      | port_label('input', 4, 'Braking Capacity')
      | port_label('input', 5, 'Vehicle Size')
      | port_label('input', 6, 'spare')
  X : Constant  Value=-72.7761459
  Y : Constant  Value=-12.4092922
  Z : Constant  Value=0
  actorID : Constant  Value=24
  actorType : Constant  Value=14
  messageID : Constant  Value=904
  rotZ : Constant  Value=180
  warning : Constant  Value=0
  Tx_Rcurve_R39 : Outport  Tag=AntennaTOutport
  wires:
  CAM generation time step/1 -> Infrastructure CAM generator/1(CAM gen time step)
  CAM repetition time step/1 -> Infrastructure CAM generator/2(CAM rep time step)
  warning/1 -> Infrastructure CAM generator/3(warning)
  messageID/1 -> Infrastructure CAM generator/4(messageID)
  actorID/1 -> Infrastructure CAM generator/5(actorID)
  actorType/1 -> Infrastructure CAM generator/6(actorType)
  Red light/1 -> Infrastructure CAM generator/7(Red light)
  Orange light/1 -> Infrastructure CAM generator/8(Orange light)
  Green light/1 -> Infrastructure CAM generator/9(Green light)
  rotZ/1 -> Infrastructure CAM generator/13(rotZ)
  X/1 -> Cartesian to GPS/1(X)
  Y/1 -> Cartesian to GPS/2(Y)
  Z/1 -> Cartesian to GPS/3(Z)
  Cartesian to GPS/1(lat) -> Infrastructure CAM generator/10(GPS_lat)
  Cartesian to GPS/2(long) -> Infrastructure CAM generator/11(GPS_long)
  Cartesian to GPS/3(alt) -> Infrastructure CAM generator/12(GPS_alt)
  Tx_Rcurve_R39_Mux/2(Out2) -> [Tx_Rcurve_R39]
  [Tx_Rcurve_R39] -> Tx_Rcurve_R39_BusAssignment/1
  Tx_Rcurve_R39_BusAssignment/1 -> Tx_Rcurve_R39_Mux/6(spare)
  Tx_Rcurve_R39_Mux/1(Out1) -> Tx_Rcurve_R39/1
  Infrastructure CAM generator/9(CAM_rotZ) -> Tx_Rcurve_R39_BusAssignment/10
  Infrastructure CAM generator/8(CAM_GPSalt) -> Tx_Rcurve_R39_BusAssignment/9
  Infrastructure CAM generator/7(CAM_GPSlong) -> Tx_Rcurve_R39_BusAssignment/8
  Infrastructure CAM generator/6(CAM_GPSlat) -> Tx_Rcurve_R39_BusAssignment/7
  Infrastructure CAM generator/5(CAM_trafficLightPriority) -> Tx_Rcurve_R39_BusAssignment/6
  Infrastructure CAM generator/4(CAM_vehicleType) -> Tx_Rcurve_R39_BusAssignment/5
  Infrastructure CAM generator/3(CAM_vehicleID) -> Tx_Rcurve_R39_BusAssignment/4
  Infrastructure CAM generator/2(CAM_messageID) -> Tx_Rcurve_R39_BusAssignment/3
  Infrastructure CAM generator/1(CAM_warning) -> Tx_Rcurve_R39_BusAssignment/2
  Ground_1/1 -> Tx_Rcurve_R39_Mux/1(warning_signal_id)
  Ground_2/1 -> Tx_Rcurve_R39_Mux/2(maneuvre_id)
  Ground_3/1 -> Tx_Rcurve_R39_Mux/3(vehicle_type)
  Ground_4/1 -> Tx_Rcurve_R39_Mux/4(brakingcapacity)
  Ground_5/1 -> Tx_Rcurve_R39_Mux/5(vehicle_size)

== system V2x_TC_IntelligentTrafficSigns_cs/TS_Rcurve_R55 ==
  CAM generation time step : Constant  Value=0.1
  CAM repetition time step : Constant  Value=0.05
  Cartesian to GPS : Subsystem
  Green light : Constant  Value=0
  Ground_{1..5} (5 alike) : Ground
  Infrastructure CAM generator : Subsystem
  Orange light : Constant  Value=0
  Red light : Constant  Value=0
  Tx_Rcurve_R55_BusAssignment : BusAssignment  Tag=BusAntennaT  AssignedSignals=warning,messageID,actorID,actorType,trafficLightPriority,GPS_lat,GPS_long,GPS_alt,rotZ
  Tx_Rcurve_R55_Mux : Subsystem  Tag=MuxAntennaT  mask(PreScan Mux Block:){Channel=1, TimeStep=1/100}
      mask prompts: TimeStep "Time Step [s]"
      settings: DeleteFcn=PreScanBlockDeleteFcn;
      mask Display:
      | fprintf('Channel: %d',Channel)
      | port_label('input', 1, 'Warning signal ID')
      | port_label('input', 2, 'Maneuvre ID')
      | port_label('input', 3, 'Vehicle Type')
      | port_label('input', 4, 'Braking Capacity')
      | port_label('input', 5, 'Vehicle Size')
      | port_label('input', 6, 'spare')
  X : Constant  Value=-72.87269
  Y : Constant  Value=-3.06407714
  Z : Constant  Value=0
  actorID : Constant  Value=27
  actorType : Constant  Value=14
  messageID : Constant  Value=904
  rotZ : Constant  Value=0
  warning : Constant  Value=0
  Tx_Rcurve_R55 : Outport  Tag=AntennaTOutport
  wires:
  CAM generation time step/1 -> Infrastructure CAM generator/1(CAM gen time step)
  CAM repetition time step/1 -> Infrastructure CAM generator/2(CAM rep time step)
  warning/1 -> Infrastructure CAM generator/3(warning)
  messageID/1 -> Infrastructure CAM generator/4(messageID)
  actorID/1 -> Infrastructure CAM generator/5(actorID)
  actorType/1 -> Infrastructure CAM generator/6(actorType)
  Red light/1 -> Infrastructure CAM generator/7(Red light)
  Orange light/1 -> Infrastructure CAM generator/8(Orange light)
  Green light/1 -> Infrastructure CAM generator/9(Green light)
  rotZ/1 -> Infrastructure CAM generator/13(rotZ)
  X/1 -> Cartesian to GPS/1(X)
  Y/1 -> Cartesian to GPS/2(Y)
  Z/1 -> Cartesian to GPS/3(Z)
  Cartesian to GPS/1(lat) -> Infrastructure CAM generator/10(GPS_lat)
  Cartesian to GPS/2(long) -> Infrastructure CAM generator/11(GPS_long)
  Cartesian to GPS/3(alt) -> Infrastructure CAM generator/12(GPS_alt)
  Tx_Rcurve_R55_Mux/2(Out2) -> [Tx_Rcurve_R55]
  [Tx_Rcurve_R55] -> Tx_Rcurve_R55_BusAssignment/1
  Tx_Rcurve_R55_BusAssignment/1 -> Tx_Rcurve_R55_Mux/6(spare)
  Tx_Rcurve_R55_Mux/1(Out1) -> Tx_Rcurve_R55/1
  Infrastructure CAM generator/9(CAM_rotZ) -> Tx_Rcurve_R55_BusAssignment/10
  Infrastructure CAM generator/8(CAM_GPSalt) -> Tx_Rcurve_R55_BusAssignment/9
  Infrastructure CAM generator/7(CAM_GPSlong) -> Tx_Rcurve_R55_BusAssignment/8
  Infrastructure CAM generator/6(CAM_GPSlat) -> Tx_Rcurve_R55_BusAssignment/7
  Infrastructure CAM generator/5(CAM_trafficLightPriority) -> Tx_Rcurve_R55_BusAssignment/6
  Infrastructure CAM generator/4(CAM_vehicleType) -> Tx_Rcurve_R55_BusAssignment/5
  Infrastructure CAM generator/3(CAM_vehicleID) -> Tx_Rcurve_R55_BusAssignment/4
  Infrastructure CAM generator/2(CAM_messageID) -> Tx_Rcurve_R55_BusAssignment/3
  Infrastructure CAM generator/1(CAM_warning) -> Tx_Rcurve_R55_BusAssignment/2
  Ground_1/1 -> Tx_Rcurve_R55_Mux/1(warning_signal_id)
  Ground_2/1 -> Tx_Rcurve_R55_Mux/2(maneuvre_id)
  Ground_3/1 -> Tx_Rcurve_R55_Mux/3(vehicle_type)
  Ground_4/1 -> Tx_Rcurve_R55_Mux/4(brakingcapacity)
  Ground_5/1 -> Tx_Rcurve_R55_Mux/5(vehicle_size)

== system V2x_TC_IntelligentTrafficSigns_cs/TS_Tcross_1 ==
  CAM generation time step : Constant  Value=0.1
  CAM repetition time step : Constant  Value=0.05
  Cartesian to GPS : Subsystem
  Green light : Constant  Value=0
  Ground_{1..5} (5 alike) : Ground
  Infrastructure CAM generator : Subsystem
  Orange light : Constant  Value=0
  Red light : Constant  Value=0
  Tx_Tcross_1_BusAssignment : BusAssignment  Tag=BusAntennaT  AssignedSignals=warning,messageID,actorID,actorType,trafficLightPriority,GPS_lat,GPS_long,GPS_alt,rotZ
  Tx_Tcross_1_Mux : Subsystem  Tag=MuxAntennaT  mask(PreScan Mux Block:){Channel=1, TimeStep=1/100}
      mask prompts: TimeStep "Time Step [s]"
      settings: DeleteFcn=PreScanBlockDeleteFcn;
      mask Display:
      | fprintf('Channel: %d',Channel)
      | port_label('input', 1, 'Warning signal ID')
      | port_label('input', 2, 'Maneuvre ID')
      | port_label('input', 3, 'Vehicle Type')
      | port_label('input', 4, 'Braking Capacity')
      | port_label('input', 5, 'Vehicle Size')
      | port_label('input', 6, 'spare')
  X : Constant  Value=-10.6195107
  Y : Constant  Value=4.51822329
  Z : Constant  Value=0
  actorID : Constant  Value=111
  actorType : Constant  Value=14
  messageID : Constant  Value=905
  rotZ : Constant  Value=0
  warning : Constant  Value=0
  Tx_Tcross_1 : Outport  Tag=AntennaTOutport
  wires:
  CAM generation time step/1 -> Infrastructure CAM generator/1(CAM gen time step)
  CAM repetition time step/1 -> Infrastructure CAM generator/2(CAM rep time step)
  warning/1 -> Infrastructure CAM generator/3(warning)
  messageID/1 -> Infrastructure CAM generator/4(messageID)
  actorID/1 -> Infrastructure CAM generator/5(actorID)
  actorType/1 -> Infrastructure CAM generator/6(actorType)
  Red light/1 -> Infrastructure CAM generator/7(Red light)
  Orange light/1 -> Infrastructure CAM generator/8(Orange light)
  Green light/1 -> Infrastructure CAM generator/9(Green light)
  rotZ/1 -> Infrastructure CAM generator/13(rotZ)
  X/1 -> Cartesian to GPS/1(X)
  Y/1 -> Cartesian to GPS/2(Y)
  Z/1 -> Cartesian to GPS/3(Z)
  Cartesian to GPS/1(lat) -> Infrastructure CAM generator/10(GPS_lat)
  Cartesian to GPS/2(long) -> Infrastructure CAM generator/11(GPS_long)
  Cartesian to GPS/3(alt) -> Infrastructure CAM generator/12(GPS_alt)
  Tx_Tcross_1_Mux/2(Out2) -> [Tx_Tcross_1]
  [Tx_Tcross_1] -> Tx_Tcross_1_BusAssignment/1
  Tx_Tcross_1_BusAssignment/1 -> Tx_Tcross_1_Mux/6(spare)
  Tx_Tcross_1_Mux/1(Out1) -> Tx_Tcross_1/1
  Infrastructure CAM generator/9(CAM_rotZ) -> Tx_Tcross_1_BusAssignment/10
  Infrastructure CAM generator/8(CAM_GPSalt) -> Tx_Tcross_1_BusAssignment/9
  Infrastructure CAM generator/7(CAM_GPSlong) -> Tx_Tcross_1_BusAssignment/8
  Infrastructure CAM generator/6(CAM_GPSlat) -> Tx_Tcross_1_BusAssignment/7
  Infrastructure CAM generator/5(CAM_trafficLightPriority) -> Tx_Tcross_1_BusAssignment/6
  Infrastructure CAM generator/4(CAM_vehicleType) -> Tx_Tcross_1_BusAssignment/5
  Infrastructure CAM generator/3(CAM_vehicleID) -> Tx_Tcross_1_BusAssignment/4
  Infrastructure CAM generator/2(CAM_messageID) -> Tx_Tcross_1_BusAssignment/3
  Infrastructure CAM generator/1(CAM_warning) -> Tx_Tcross_1_BusAssignment/2
  Ground_1/1 -> Tx_Tcross_1_Mux/1(warning_signal_id)
  Ground_2/1 -> Tx_Tcross_1_Mux/2(maneuvre_id)
  Ground_3/1 -> Tx_Tcross_1_Mux/3(vehicle_type)
  Ground_4/1 -> Tx_Tcross_1_Mux/4(brakingcapacity)
  Ground_5/1 -> Tx_Tcross_1_Mux/5(vehicle_size)
